simulink model "MPC_gamecontroller"  (Simulink 7.6)
settings: Solver=ode3  StartTime=0.0  StopTime=inf  FixedStep=.05  RelTol=1e-3
InitFcn:
  | a2g = 1/9.81;
  | r2d = 180/pi;
  | K_sr = 15.97;
  | neutral_steer = 103;
  | s2t = (pi/180)*(1/K_sr);
  | A_kt =[         0         0         0         0         0         0
  |          0   -3.5058   -1.6990  -25.6114   -2.2435         0
  |          0    2.2782   -4.2575  -64.1772   -5.6218         0
  |          0         0    1.0000         0         0         0
  |          0   -0.0158    0.0295    0.4442   -4.1900         0
  |          0         0         0         0    1.0000         0];
  | 
  | 
  | B_kt =[0
  |    23.7845
  |   -16.0342
  |          0
  |    17.5770
  |          0];
  | 
  | dt = 0.05;
  | measnoise_posX = 3.36;
  | measnoise_posY = 3.95;
  | measnoise_theta = 0.136;
  | measnoise_phi =  0.078;
  | measnoise_velocity = measnoise_posX*dt;
  | 
  | accelnoiseX = 0.97;
  | accelnoiseY = 1.36;
  | gyronoisetheta = 0.04;
  | gyronoisephi = 0.021;
  | 
  | anx = accelnoiseX^2;
  | any = accelnoiseY^2;
  | anxy = accelnoiseX*accelnoiseY;
  | gntheta = gyronoisetheta^2;
  | agthetax = gyronoisetheta*accelnoiseX;
  | agthetay = gyronoisetheta*accelnoiseY;
  | gnphi = gyronoisephi^2;
  | agphix = gyronoisephi*accelnoiseX;
  | agphiy = gyronoisephi*accelnoiseY;
  | gnthetaphi = gyronoisetheta*gyronoisephi;
  | 
  | A = [1 dt 0 0 0 0;...
  |         0 1 0 0 0 0;...
  |         0 0 1 dt 0 0;...
  |         0 0 0 1 0 0;...
  |         0 0 0 0 1 0;...
  |         0 0 0 0 0 1];
  | 
  | Sw = [anx*dt^4/4, anx*dt^3/2, anxy*dt^4/4, anxy*dt^3/2,  agthetax*dt^3/2, agphix*dt^3/2;...
  |       anx*dt^3/2, anx*dt^2,   anxy*dt^3/2, anxy*dt^2, agthetax*dt^2,   agphix*dt^2;...
  |       anxy*dt^4/4, anxy*dt^3/2, any*dt^4/4, any*dt^3/2,  agthetay*dt^3/2, agphiy*dt^3/2;...
  |       anxy*dt^3/2, anxy*dt^2,   any*dt^3/2, any*dt^2, agthetay*dt^2, agphiy*dt^2;...
  |       agthetax*dt^3/2, agthetax*dt^2, agthetay*dt^3/2, agthetay*dt^2, gntheta*dt^2, gnthetaphi*dt^2;...
  |       agphix*dt^3/2, agphix*dt^2, agphiy*dt^3/2, agphiy*dt^2, gnthetaphi*dt^2, gnphi*dt^2];
  | 
  | Sz =  [measnoise_posX^2, 0, 0, 0, 0, 0;...
  |          0, measnoise_velocity^2, 0, 0, 0, 0;...
  |          0, 0, measnoise_posY^2, 0, 0, 0;...
  | ... (+5 lines)
StopFcn:
  | %x = x_values.signals.values;
  | %y = -y_values.signals.values;
  | %yL = y + 0.5;
  | %yR = y - 0.5;
  | 
  | %hold all;
  | 
  | %plot(x,yL,'r');
  | %plot(x,yR,'r');
  | 
  | %line([15 17],[0 0]);
  | %line([15 17],[-1 -1]);
  | %line([15 15],[0 -1]);
  | %line([17 17],[0 -1]);
  | %axis([0 30 -5 5]);
  | 
  | %hold off;
undefined symbols (not defined in the file or in its folder): CR, DiscIntNeverNeededParam, fdesignblkfcn, gcbh, serial
libraries: simulink x417, instrumentlib x3, dspadpt3 x1, realtime_pacer_lib x1, vrlib x1
(part 3 of 3)

== matlab function Steering Wheel Control/MATLAB Function ==
  data: direction input double; steer output double; throttle input double; neutral input double; kill input double; last_state input double
function steer = controller_to_steer(direction,throttle,neutral,kill,last_state)
%#codegen
if(kill)
   steer = neutral;
else 
 steer = last_state;
 steer(2) = direction;
 steer(3) = throttle;   
end

== matlab function Data Sorting/Embedded MATLAB Function ==
  data: old_state input double; roll input double; yaw input double; wheel_speeds input double; RRT_state output double; MPC_state output double
% function [x,y,theta,velocity, TESTING] = fcn(x_old,y_old,theta_old,yaw_rate_IMU,Vl_old,Vr_old)
% %#eml
% 
% deltaK = 0.05;
% 
% delta_theta_enc = (Vr_old - Vl_old)*(12/10)*deltaK;
% delta_theta_imu = yaw_rate_IMU*deltaK;
% delta_theta = (delta_theta_enc+delta_theta_imu)/2;
% 
% theta = theta_old + delta_theta;
% 
% velocity = (Vl_old + Vr_old)/2;
% 
% x = x_old + velocity*-1*sin(theta_old)*deltaK;
% y = y_old + velocity*cos(theta_old)*deltaK;

%NEW AWESOME DATA SORTING
function [RRT_state,MPC_state]  = data_sorting(old_state,roll,yaw, wheel_speeds)
%#eml
deltaK = 0.05;

x_old = old_state(1);
y_old = old_state(2);

velocity = (wheel_speeds(1) + wheel_speeds(2) + wheel_speeds(3) + wheel_speeds(4))/4;

Phi_d = roll(1);
Phi = roll(2);
Psi_d = yaw(1);
Psi = yaw(2);
Vx = velocity*sin(Psi);
Vy = velocity*cos(Psi); 

x = x_old - Vx*deltaK;
y = y_old + Vy*deltaK;

MPC_state = [Vx*(1/3.28), Vy*(1/3.28), Phi_d, Phi, Psi_d, Psi]';
RRT_state = [x, y, Psi, velocity]';

== matlab function Steering Wheel Control/MATLAB Function1 ==
  data: angler input double; turnangle output double
function turnangle = scale_steer(angler)
%#codegen
if(angler>0)
    turnangle = 100+angler*(255-100);
elseif(angler<0)
    turnangle = 100+angler*100;
else 
    turnangle = 100;
end

== matlab function Steering Wheel Control/MATLAB Function2 ==
  data: pedal input double; speed output double
function speed = scale_throttle(pedal)
%#codegen
if(pedal>0)
    speed = 100-pedal*100;
elseif(pedal<0)
     speed = 100-pedal*(255-100);
else 
    speed = 100;
end
